simulink model "WheelLoads"  (R2020b)
settings: Solver=ode23  StartTime=0.0  StopTime=5  RelTol=0.01  AbsTol=0.01
PreLoadFcn:
  | addpath('Functions');
  | addpath('Functions/geometric');
  | addpath('Parameters');
  | vhclParams;
  | 
  | ELLIPSE = Simulink.Variant('variantMode == 1');
  | CONSTANT_ACC = Simulink.Variant('variantMode == 2');
  | CONSTANT_OUT = Simulink.Variant('variantMode == 3');
  | EXTERNAL_DATA_LOAD = Simulink.Variant('variantMode == 4');
  | EXTERNAL_DATA_ACC = Simulink.Variant('variantMode == 5');
  | if ~exist('F','var')
  |  F = 0;
  | end
  | if ~exist('M','var')
  |  M = 0;
  | end
  | if ~exist('St','var')
  |  St = 0;
  | end
  | if ~exist('Ax','var')
  |  Ax = 0;
  | end
  | if ~exist('Ay','var')
  |  Ay = 0;
  | end
  | if ~exist('StAcc','var')
  |  StAcc = 0;
  | end
InitFcn:
  | [folder, ~, ~] = fileparts(which (bdroot));
  | cd(folder);
undefined symbols (not defined in the file): ARB_bcrnk_frontL, ARB_bcrnk_frontR, ARB_bcrnk_rearL, ARB_bcrnk_rearR, ARB_center_front, ARB_center_rear, ARB_damping_front, ARB_damping_rear, ARB_length_front, ARB_length_rear, ARB_pinSlot_correctionFactor_front, ARB_pinSlot_correctionFactor_rear, ARB_stiffness_front, ARB_stiffness_rear, ARB_TieRod_length_front, ARB_TieRod_length_rear, COG, Damper_coefficient_front, Damper_coefficient_rear, Driver_COG, Driver_mass, DriveTrain_ratio, FS_camber, FS_toe, gravity, Mass, Rim_mass, RodDiameter, RodThickness, RS_camber, RS_toe, sep_lower_front, sep_lower_rear, sep_upper_front, sep_upper_rear, Spring_preload_front, Spring_preload_rear, Spring_stiffness_front, Spring_stiffness_rear, Sprung_mass, Sprung_mass_COG, sym_lower_front, sym_lower_rear, sym_upper_front, sym_upper_rear, Tire_mass, Tire_radius, Unsprung_mass_COG_front, Unsprung_mass_COG_rear, Unsprung_mass_front, Unsprung_mass_rear, util_rodCrossSection, Wheel_inertia, X, X_F, X_R, Y, Z
libraries: sm_lib x400, nesl_utility x70, fl_lib x1
(part 2 of 18)

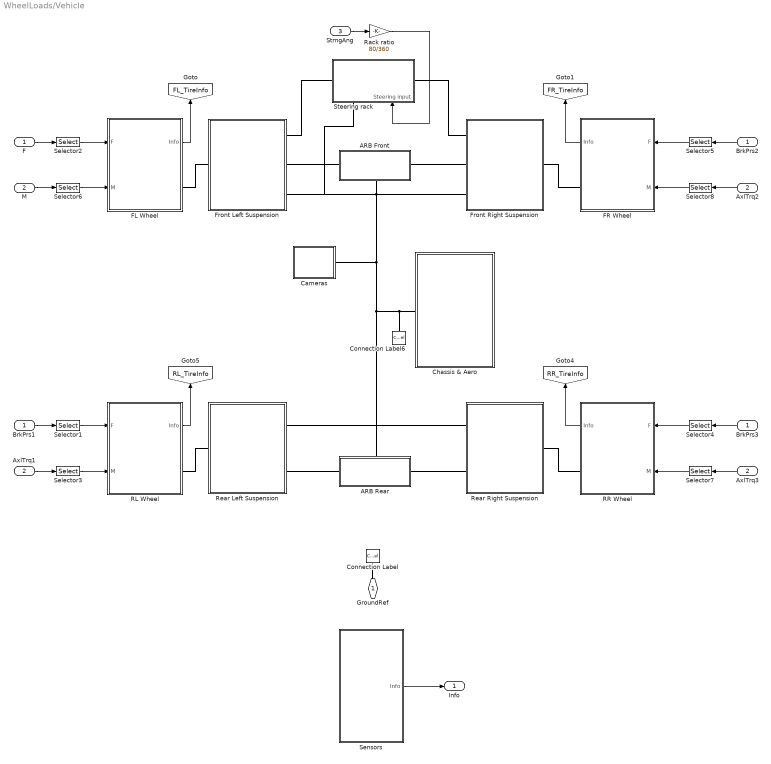
== system WheelLoads/Vehicle ==
  F : Inport
  M : Inport  Port=2
  StrngAng : Inport  Port=3
  ARB Front : Subsystem  mask{arbStiffness=ARB_stiffness_front, arbDamp=ARB_damping_front, arbCorrection=ARB_pinSlot_correctionFactor_front, arbCenter=ARB_center_front, arbLength=ARB_length_front, arbRodLength=ARB_TieRod_length_front, RodDiam=RodDiameter, RodThick=RodThickness}
      mask prompts: arbStiffness "Anti-roll Bar Stiffness"; arbDamp "Anti-roll Bar Damping"; arbCorrection "Stiffness correction factor"; arbCenter "Anti-roll Bar center"; arbLength "Anti-roll Bar length"; arbRodLength "Anti-roll Bar tie-rod length"; RodDiam "Rod diameter"; RodThick "Rod thickness"
      settings: PortSchema="{\"entries\":[{\"content\":{\"sides\":[{\"content\":{\"connectorIds\":[\"LConn1\"]},\"type\":\"ConnectorPlacement.EquallySpacedRectSide\",\"uuid\":\"7b39e7e2-1c99-4477-9d88-8155f0dcbcfa\"},{\"content\":{\"connectorIds\":[\"RConn1\"],\"side\":\"RIGHT\"},\"type\":\"ConnectorPlacement.EquallySpacedRectSide\",\"uuid\":\"e62feb70-34ca-4fb6-b792-0423ade04344\"},{\"content\":{\"connectorIds\":[],\"side\":\"TOP\"},\"type\":\"ConnectorPlacement.EquallySpacedRectSide\",\"uuid\":\"b26fb64d-892c-44a6-8f06-11f439d11cb6\"},{\"content\":{\"connectorIds\":[\"RConn2\"],\"side\":\"BOTTOM\"},\"type\":\"ConnectorPlacement.EquallySpacedRectSide\",\"uuid\":\"e4489596-2281-46d7-9d2e-4ef3bf65c061\"}]},\"type\":\"ConnectorPlacement.EquallySpacedConnector\",\"uuid\":\"4e7c908e-7a44-4075-b019-e55e6c824031\"}],\"packageUris\":[\"http://schema.mathworks.com/mf0/Connector/19700101\"],\"version\":\"1.0\"}"
      mask DialogControl DescGroupVar Prompt: Anti-roll Bar
      mask DialogControl DescTextVar Prompt: Z-Type anti-roll bar assembly
      mask DialogControl ParameterGroupVar Prompt: Dynamics
      mask DialogControl arbStiffness Tooltip: Anti-roll bar stiffness (N/mm)
      mask DialogControl arbDamp Tooltip: Anti-roll bar damping (N/(mm/s))
      mask DialogControl Container4 Prompt: Geometry
      mask DialogControl arbCenter Tooltip: Coordinates of the anti-roll bar center (mm)
      mask DialogControl arbLength Tooltip: Length of the anti-roll bar arm (mm)
      mask DialogControl arbRodLength Tooltip: length of the actuation rod of the anti-roll bar (mm)
      mask DialogControl Container5 Prompt: Graphic
      mask Display: image('$imagefile'); % Use "Edit Image" on toolstrip to change.
  ARB Rear : Subsystem  mask{arbStiffness=ARB_stiffness_rear, arbDamp=ARB_damping_rear, arbCorrection=ARB_pinSlot_correctionFactor_rear, arbCenter=ARB_center_rear, arbLength=ARB_length_rear, arbRodLength=ARB_TieRod_length_rear, RodDiam=RodDiameter, RodThick=RodThickness}
      mask prompts: arbStiffness "Anti-roll Bar Stiffness"; arbDamp "Anti-roll Bar Damping"; arbCorrection "Stiffness correction factor"; arbCenter "Anti-roll Bar center"; arbLength "Anti-roll Bar length"; arbRodLength "Anti-roll Bar tie-rod length"; RodDiam "Rod diameter"; RodThick "Rod thickness"
      settings: PortSchema="{\"entries\":[{\"content\":{\"sides\":[{\"content\":{\"connectorIds\":[\"LConn1\"]},\"type\":\"ConnectorPlacement.EquallySpacedRectSide\",\"uuid\":\"7b39e7e2-1c99-4477-9d88-8155f0dcbcfa\"},{\"content\":{\"connectorIds\":[\"RConn1\"],\"side\":\"RIGHT\"},\"type\":\"ConnectorPlacement.EquallySpacedRectSide\",\"uuid\":\"e62feb70-34ca-4fb6-b792-0423ade04344\"},{\"content\":{\"connectorIds\":[],\"side\":\"TOP\"},\"type\":\"ConnectorPlacement.EquallySpacedRectSide\",\"uuid\":\"b26fb64d-892c-44a6-8f06-11f439d11cb6\"},{\"content\":{\"connectorIds\":[\"RConn2\"],\"side\":\"BOTTOM\"},\"type\":\"ConnectorPlacement.EquallySpacedRectSide\",\"uuid\":\"e4489596-2281-46d7-9d2e-4ef3bf65c061\"}]},\"type\":\"ConnectorPlacement.EquallySpacedConnector\",\"uuid\":\"4e7c908e-7a44-4075-b019-e55e6c824031\"}],\"packageUris\":[\"http://schema.mathworks.com/mf0/Connector/19700101\"],\"version\":\"1.0\"}"
      mask DialogControl DescGroupVar Prompt: Anti-roll Bar
      mask DialogControl DescTextVar Prompt: Z-Type anti-roll bar assembly
      mask DialogControl ParameterGroupVar Prompt: Dynamics
      mask DialogControl arbStiffness Tooltip: Anti-roll bar stiffness (N/mm)
      mask DialogControl arbDamp Tooltip: Anti-roll bar damping (N/(mm/s))
      mask DialogControl Container4 Prompt: Geometry
      mask DialogControl arbCenter Tooltip: Coordinates of the anti-roll bar center (mm)
      mask DialogControl arbLength Tooltip: Length of the anti-roll bar arm (mm)
      mask DialogControl arbRodLength Tooltip: length of the actuation rod of the anti-roll bar (mm)
      mask DialogControl Container5 Prompt: Graphic
      mask Display: image('$imagefile'); % Use "Edit Image" on toolstrip to change.
  AxlTrq1 : InportShadow  Port=2
  AxlTrq2 : InportShadow  Port=2
  AxlTrq3 : InportShadow  Port=2
  BrkPrs1 : InportShadow
  BrkPrs2 : InportShadow
  BrkPrs3 : InportShadow
  Cameras : Subsystem
      mask Display: image('$imagefile'); % Use "Edit Image" on toolstrip to change.
  Chassis & Aero : Subsystem
      settings: PortSchema="{\"entries\":[{\"content\":{\"sides\":[{\"content\":{\"connectorIds\":[]},\"type\":\"ConnectorPlacement.EquallySpacedRectSide\",\"uuid\":\"cbbe6af3-19fb-48b3-8800-b215223b12f6\"},{\"content\":{\"connectorIds\":[],\"side\":\"RIGHT\"},\"type\":\"ConnectorPlacement.EquallySpacedRectSide\",\"uuid\":\"e0ce8cb6-c1e3-4be9-8f24-6f1308fe5f38\"},{\"content\":{\"connectorIds\":[\"LConn1\"],\"side\":\"TOP\"},\"type\":\"ConnectorPlacement.EquallySpacedRectSide\",\"uuid\":\"4e525be5-d309-4877-ab04-513b663981dd\"},{\"content\":{\"connectorIds\":[],\"side\":\"BOTTOM\"},\"type\":\"ConnectorPlacement.EquallySpacedRectSide\",\"uuid\":\"1a8d8adb-4793-4ada-a6b7-ecb65b96140c\"}]},\"type\":\"ConnectorPlacement.EquallySpacedConnector\",\"uuid\":\"0639ed7d-0262-43c4-ade7-52e76f974124\"}],\"packageUris\":[\"http://schema.mathworks.com/mf0/Connector/19700101\"],\"version\":\"1.0\"}"
      mask Display:
      | image('$imagefile'); % Use "Edit Image" on toolstrip to change.
      | port_label('LConn',1,'Ref')
  Connection Label : ConnectionLabel  Label=GroundRef
  Connection Label6 : ConnectionLabel  Label=GroundRef
  FL Wheel : Subsystem  mask{Wheel_mass=Rim_mass+Tire_mass, Wheel_inertia=Wheel_inertia, side=Left, sideSign=1}
      mask prompts: Wheel_mass "Wheel Mass"; Wheel_inertia "Wheel Inertia"
      mask TypeOptions side: Left | Right
      mask Callback side:
      | switch get_param(gcb,'side')
      |     case 'Left'
      |         set_param(gcb,'sideSign','1');
      |     case 'Right'
      |         set_param(gcb,'sideSign','-1');
      | end
      mask Display: image('$imagefile'); % Use "Edit Image" on toolstrip to change.
  FR Wheel : Subsystem  mask{Wheel_mass=Rim_mass+Tire_mass, Wheel_inertia=Wheel_inertia, side=Right, sideSign=-1}
      mask prompts: Wheel_mass "Wheel Mass"; Wheel_inertia "Wheel Inertia"
      mask TypeOptions side: Left | Right
      mask Callback side:
      | switch get_param(gcb,'side')
      |     case 'Left'
      |         set_param(gcb,'sideSign','1');
      |     case 'Right'
      |         set_param(gcb,'sideSign','-1');
      | end
      mask Display: image('$imagefile'); % Use "Edit Image" on toolstrip to change.
  Front Left Suspension : Subsystem  mask{X_original=X_F, X=[1090.2 243.1 189 810.3 257.6 186.1 1026.7 182.2 32.9 815.9 185.3 32.8 950.3 506 220 963.9 512 29 1090 225 42 1010 535 41 957 600 128.6 0 0.998629534754574 0.0523359562429438 950.3 487.586 237.194 955.54 278.351 490.117 951.389 225.774 445.625 953.389 219.438 514.305 950.3 40 499], enOptim=off, sepUpper=sep_upper_front, symUpper=sym_upper_front, sepLower=sep_lower_front, symLower=sym_lower_front, side=Left, sideSign=1, ARB_bcrnk=ARB_bcrnk_frontL, Camber=FS_camber, Toe=FS_toe, BcrnkPos=0, DamperPos=[0 90 0], ARB_On=on, St_On=on, SpringStiffness=Spring_stiffness_front, SpringPreload=Spring_preload_front, DamperCoef=Damper_coefficient_front, DamperMaxExt=200, DampLimit_MaxK=10000, DampLimit_MaxC=1, DampLimit_MaxTrans=1, DamperMinExt=154, DampLimit_MinK=2000, DampLimit_MinC=1, DampLimit_MinTrans=5, PushSetupLength=0, ToeSetupLength=0, UnsprungMass=Unsprung_mass_front-Tire_mass-Rim_mass, UnsprungMassCOG=Unsprung_mass_COG_front, RodThick=1, RodDiam=14, UCAfore_X=152.984798840791, UCAaft_X=-127.305532779834, UCA_Y=-257.3812, UCA_pushBracket_X=1.13049065291656, UCA_pushBracket_Y=-16.0659716079757, UCA_pushBracket_Z=19.373063236757, LCAfore_X=67.6445283762746, LCAaft_X=-143.178288225724, LCA_Y=-328.86366138683, UCA_Rot=[0.0129952164868874 0.0511310087922121 0.998607402480249;0.0511310087922121 0.997351198186899 -0.0517320733689305;-0.998607402480249 0.0517320733689305 0.0103464146737861], UCAfore_Rot=[-0.883968661732343 -0.0167990787372824 -0.467244257352307;-0.461720605527559 -0.125900009513931 0.878045141228887;-0.0735764059083774 0.991900689866667 0.103535182115236], UCAaft_Rot=[-0.872927868673978 -0.0167990787372824 0.48755997276836;0.485594358546025 -0.125900009513931 0.865070694540433;0.0468514144992482 0.991900689866667 0.118059164834624], LCA_Rot=[0.000690445618848567 0.0146957287408994 0.99989177356433;0.0146957287408994 0.999783886342046 -0.0147042907877108;-0.99989177356433 0.0147042907877108 0.000474331960894192], LCAfore_Rot=[-0.982351350121625 -0.000301304030408464 -0.187044738311724;-0.187035279606844 0.0117671555848941 0.982282718076538;0.00190501879509281 0.999930719232749 -0.011615835659486], LCAaft_Rot=[-0.91090135192941 -0.000301304030408464 0.412624085905142;0.412592306855799 0.0117671555848941 0.910839789629796;-0.00512985151659236 0.999930719232749 -0.0105944022056725], BcrnkDamp=69.0006325768104, BcrnkPush=69.0007956041088, BcrnkAng=54.9995482296285, BcrnkRot=[-0.0310893815864683 0.0519417534127774 0.998166070654066;0.0519417534127774 0.997383402646003 -0.0502832216418927;-0.998166070654066 0.0502832216418927 -0.0337059789404655], PushRot=[0.998890321638943 0.0443098381442263 -0.0159613150987419;0.0443098381442263 -0.769307058035718 0.637340794787264;0.0159613150987419 -0.637340794787264 -0.770416736396775], SteeringRot=[0.937365949814546 0.242706944468636 -0.249876800113523;0.242706944468636 0.0595105901840355 0.9682726004399;0.249876800113523 -0.9682726004399 -0.00312346000141894], Parameter55=on, Parameter56=0}
      mask prompts: X_original "Coordinates"; X "Coordinates_"; enOptim "Enable"; sepUpper "Separation"; symUpper "Symmetry"; sepLower "Separation"; symLower "Symmety"; ARB_bcrnk "ARB tie-rod"; BcrnkPos "Bellcrank initial rotation"; DamperPos "Damper initial position"; ARB_On "Anti-roll bar"; St_On "Steering"; SpringStiffness "Spring stiffness"; SpringPreload "Spring preload"; DamperCoef "Damping Coefficient"; DamperMaxExt "Maximum extension"; DampLimit_MaxK "Max_Stiffness"; DampLimit_MaxC "Max_Damping"; DampLimit_MaxTrans "Max_Transition"; DamperMinExt "Maximum compression"; DampLimit_MinK "Min_Stiffness"; DampLimit_MinC "Min_Damping"; DampLimit_MinTrans "Min_Transition"; PushSetupLength "Push"; ToeSetupLength "Toe"; UnsprungMass "Mass"; UnsprungMassCOG "COG"; RodThick "Rod Thickness"; RodDiam "Rod Diameter"
      mask help: Define a Simscape Multibody model of a double control arm independent suspension
      mask init:
      | dblAarmSuspOptim_init(gcb)
      mask Help:
      | -Geometry:
      |     +Suspension Coordinates: coordinates of the points that define a double wishbone suspension system. The coordinates must always correspond to the LEFT side. The variable (e.g. 'X') containing the data must be a row vector with the following values, in order:
      |       1-A-Arm Upper Front hardpoint. X([1 2 3])
      |       2-A-Arm Upper Rear. X([4 5 6])
      |       3-A-Arm Lower Front. X([7 8 9])
      |       4-A-Arm Lower Rear. X([10 11 12])
      |       5-A-Arm Upper Upright. X([13 14 15])
      |       6-A-Arm Lower Upright. X([16 17 18])
      |       7-Steering Rack. X([19 20 21])
      |       8-Steering Upright. X([22 23 24])
      |       9-Wheel Center. X([25 26 27])
      |       10-Wheel Plane normal vector. X([28 29 30])
      |       11-A-Arm Push Bracket. X([31 32 33])
      |       12-Bellcrank Push Bracket. X([34 35 36])
      |       13-Bellcrank Chassis Bracket. X([37 38 39])
      |       14-Bellcrank Damper Bracket. X([40 41 42])
      |       15-Damper Bracket. X([43 44 45])
      |       16-Bellcrank ARB Bracket. X(46:48)
      |       17-ARB end. X(49:51)
      |       18-ARB center. X(52:54)
      |     +It must be noted that in the model the bellcranks have the same dimensions as defined in the geometry, but the position and orientation is diferent. It is positioned in a vertical plane that contains the a-arm push bracket. The reason is to let the anti-roll bar be symmetric.
      mask TypeOptions side: Left | Right
      mask Callback side:
      | switch get_param(gcb,'side')
      |     case 'Left'
      |         set_param(gcb,'sideSign','1');
      |     case 'Right'
      |         set_param(gcb,'sideSign','-1');
      | end
      mask DialogControl DescGroupVar Prompt: Double Wishbone Suspension
      mask DialogControl SuspGeomTab Prompt: Geometry
      mask DialogControl X_original Tooltip: Variable containting suspension points coordinates
      mask DialogControl Container25 Prompt: Modify a-arms geometry
      mask DialogControl enOptim Tooltip: Below parameters are used to modify the provided geometry. In this enable button is off no changes will be made
      mask DialogControl Container26 Prompt: Upper arm
      mask DialogControl sepUpper Tooltip: Separation between a-arm hardpoints (mm)
      mask DialogControl symUpper Tooltip: Symmetry factor. Value between -1 and 1. Use 0 for perfectly symmetric arm
      mask DialogControl Container27 Prompt: Lower arm
      mask DialogControl sepLower Tooltip: Separation between a-arm hardpoints (mm)
      mask DialogControl symLower Tooltip: Symmetry factor. Value between -1 and 1. Use 0 for perfectly symmetric arm
      mask DialogControl Container22 Prompt: Anti-roll Bar
      mask DialogControl ARB_bcrnk Tooltip: Coordinates of the attachment point of the ARB to the bellcrank (mm)
      mask DialogControl Container8 Prompt: Wheel angles
      mask DialogControl Camber Tooltip: Camber angle in deg at reference ride height
      mask DialogControl Toe Tooltip: Toe angle in deg at reference ride height
      mask DialogControl Container23 Prompt: Assembly
      mask DialogControl BcrnkPos Tooltip: Degrees of rotation of follower frame respect to base frame of the bellcrank bracket joint for the inital position
      mask DialogControl DamperPos Tooltip: Degrees of rotation of follower frame respect to base frame of the bellcrank bracket joint for the inital position, specified as a rotation sequence X-Y-Z
      mask DialogControl ARB_On Tooltip: Select wheter the block displays connection for ARB or not
      mask DialogControl St_On Tooltip: Select wether the block displays steering input or not
      mask DialogControl SpringDampTab Prompt: Spring and Damper
      mask DialogControl SpringStiffness Tooltip: Spring stiffness (lb/inch)
      mask DialogControl SpringPreload Tooltip: Spring preload in mm
      mask DialogControl DamperCoef Tooltip: Damping Coefficient in N/(mm/s)
      mask DialogControl Container9 Prompt: Limits
      mask DialogControl DamperMaxExt Tooltip: Maximum allowable extension of the damper, meassured between ball-joints. This value is also used for the natural length of the spring (mm)
      mask DialogControl DampLimit_MaxK Tooltip: Stiffness when upper limit is reached in N/mm
      mask DialogControl DampLimit_MaxC Tooltip: Damping coefficient when upper limit is reached in N/(mm/s)
      mask DialogControl DampLimit_MaxTrans Tooltip: Transition region width between normal behavior and limit
      mask DialogControl DamperMinExt Tooltip: Minimum allowable extension (max compression) of the damper, meassured between ball-joints (mm)
      mask DialogControl DampLimit_MinK Tooltip: Stiffness when lower limit is reached in N/(mm/s)
      mask DialogControl DampLimit_MinC Tooltip: Damping coefficient when lower limit is reached in N/(mm/s)
      mask DialogControl DampLimit_MinTrans Tooltip: Transition region width between normal behavior and limit
      mask DialogControl Container13 Prompt: Setup
      mask DialogControl PushSetupLength Tooltip: Length adjustment of the rod ends in the push rod
      mask DialogControl ToeSetupLength Tooltip: Length adjustment of the rod ends in the toe/steering rod
      mask DialogControl Container19 Prompt: Mass
      mask DialogControl Container20 Prompt: Non-rotating unsprung mass
      mask DialogControl UnsprungMass Tooltip: Non rotating unsprung mass (kg)
      mask DialogControl UnsprungMassCOG Tooltip: Non-rotating unsprung mass center of gravity coordinates (mm)
      mask DialogControl Container17 Prompt: Graphic
      mask DialogControl internalParamContainer Prompt: Internal params
      mask Display: image('$imagefile'); % Use "Edit Image" on toolstrip to change.
  Front Right Suspension : Subsystem  mask{X_original=X_F, X=[1090.2 243.1 189 810.3 257.6 186.1 1026.7 182.2 32.9 815.9 185.3 32.8 950.3 506 220 963.9 512 29 1090 225 42 1010 535 41 957 600 128.6 0 0.998629534754574 0.0523359562429438 950.3 487.586 237.194 955.54 278.351 490.117 951.389 225.774 445.625 953.389 219.438 514.305 950.3 40 499], enOptim=off, sepUpper=sep_upper_front, symUpper=sym_upper_front, sepLower=sep_lower_front, symLower=sym_lower_front, side=Right, sideSign=-1, ARB_bcrnk=ARB_bcrnk_frontR, Camber=FS_camber, Toe=FS_toe, BcrnkPos=0, DamperPos=[0 90 0], ARB_On=on, St_On=on, SpringStiffness=Spring_stiffness_front, SpringPreload=Spring_preload_front, DamperCoef=Damper_coefficient_front, DamperMaxExt=200, DampLimit_MaxK=10000, DampLimit_MaxC=1, DampLimit_MaxTrans=1, DamperMinExt=154, DampLimit_MinK=2000, DampLimit_MinC=1, DampLimit_MinTrans=5, PushSetupLength=0, ToeSetupLength=0, UnsprungMass=Unsprung_mass_front-Tire_mass-Rim_mass, UnsprungMassCOG=Unsprung_mass_COG_front, RodThick=1, RodDiam=14, UCAfore_X=152.984798840791, UCAaft_X=-127.305532779834, UCA_Y=-257.3812, UCA_pushBracket_X=1.13049065291656, UCA_pushBracket_Y=-16.0659716079757, UCA_pushBracket_Z=19.373063236757, LCAfore_X=67.6445283762746, LCAaft_X=-143.178288225724, LCA_Y=-328.86366138683, UCA_Rot=[0.0129952164868874 -0.0511310087922121 0.998607402480249;-0.0511310087922121 0.997351198186899 0.0517320733689305;-0.998607402480249 -0.0517320733689305 0.0103464146737861], UCAfore_Rot=[-0.883968661732343 0.0167990787372824 -0.467244257352307;0.461720605527559 -0.125900009513931 -0.878045141228887;-0.0735764059083774 -0.991900689866667 0.103535182115236], UCAaft_Rot=[-0.872927868673978 0.0167990787372824 0.48755997276836;-0.485594358546025 -0.125900009513931 -0.865070694540433;0.0468514144992482 -0.991900689866667 0.118059164834624], LCA_Rot=[0.000690445618848567 -0.0146957287408994 0.99989177356433;-0.0146957287408994 0.999783886342046 0.0147042907877108;-0.99989177356433 -0.0147042907877108 0.000474331960894192], LCAfore_Rot=[-0.982351350121625 0.000301304030408464 -0.187044738311724;0.187035279606844 0.0117671555848941 -0.982282718076538;0.00190501879509281 -0.999930719232749 -0.011615835659486], LCAaft_Rot=[-0.91090135192941 0.000301304030408464 0.412624085905142;-0.412592306855799 0.0117671555848941 -0.910839789629796;-0.00512985151659236 -0.999930719232749 -0.0105944022056725], BcrnkDamp=69.0006325768104, BcrnkPush=69.0007956041088, BcrnkAng=54.9995482296285, BcrnkRot=[-0.0310893815864683 -0.0519417534127774 0.998166070654066;-0.0519417534127774 0.997383402646003 0.0502832216418927;-0.998166070654066 -0.0502832216418927 -0.0337059789404655], PushRot=[0.998890321638943 -0.0443098381442263 -0.0159613150987419;-0.0443098381442263 -0.769307058035718 -0.637340794787264;0.0159613150987419 0.637340794787264 -0.770416736396775], SteeringRot=[0.937365949814546 -0.242706944468636 -0.249876800113523;-0.242706944468636 0.0595105901840355 -0.9682726004399;0.249876800113523 0.9682726004399 -0.00312346000141894]}
      mask prompts: X_original "Coordinates"; X "Coordinates_"; enOptim "Enable"; sepUpper "Separation"; symUpper "Symmetry"; sepLower "Separation"; symLower "Symmety"; ARB_bcrnk "ARB tie-rod"; BcrnkPos "Bellcrank initial rotation"; DamperPos "Damper initial position"; ARB_On "Anti-roll bar"; St_On "Steering"; SpringStiffness "Spring stiffness"; SpringPreload "Spring preload"; DamperCoef "Damping Coefficient"; DamperMaxExt "Maximum extension"; DampLimit_MaxK "Max_Stiffness"; DampLimit_MaxC "Max_Damping"; DampLimit_MaxTrans "Max_Transition"; DamperMinExt "Maximum compression"; DampLimit_MinK "Min_Stiffness"; DampLimit_MinC "Min_Damping"; DampLimit_MinTrans "Min_Transition"; PushSetupLength "Push"; ToeSetupLength "Toe"; UnsprungMass "Mass"; UnsprungMassCOG "COG"; RodThick "Rod Thickness"; RodDiam "Rod Diameter"
      mask help: Define a Simscape Multibody model of a double control arm independent suspension
      mask init:
      | dblAarmSuspOptim_init(gcb)
      mask Help:
      | -Geometry:
      |     +Suspension Coordinates: coordinates of the points that define a double wishbone suspension system. The coordinates must always correspond to the LEFT side. The variable (e.g. 'X') containing the data must be a row vector with the following values, in order:
      |       1-A-Arm Upper Front hardpoint. X([1 2 3])
      |       2-A-Arm Upper Rear. X([4 5 6])
      |       3-A-Arm Lower Front. X([7 8 9])
      |       4-A-Arm Lower Rear. X([10 11 12])
      |       5-A-Arm Upper Upright. X([13 14 15])
      |       6-A-Arm Lower Upright. X([16 17 18])
      |       7-Steering Rack. X([19 20 21])
      |       8-Steering Upright. X([22 23 24])
      |       9-Wheel Center. X([25 26 27])
      |       10-Wheel Plane normal vector. X([28 29 30])
      |       11-A-Arm Push Bracket. X([31 32 33])
      |       12-Bellcrank Push Bracket. X([34 35 36])
      |       13-Bellcrank Chassis Bracket. X([37 38 39])
      |       14-Bellcrank Damper Bracket. X([40 41 42])
      |       15-Damper Bracket. X([43 44 45])
      |       16-Bellcrank ARB Bracket. X(46:48)
      |       17-ARB end. X(49:51)
      |       18-ARB center. X(52:54)
      |     +It must be noted that in the model the bellcranks have the same dimensions as defined in the geometry, but the position and orientation is diferent. It is positioned in a vertical plane that contains the a-arm push bracket. The reason is to let the anti-roll bar be symmetric.
      mask TypeOptions side: Left | Right
      mask Callback side:
      | switch get_param(gcb,'side')
      |     case 'Left'
      |         set_param(gcb,'sideSign','1');
      |     case 'Right'
      |         set_param(gcb,'sideSign','-1');
      | end
      mask DialogControl DescGroupVar Prompt: Double Wishbone Suspension
      mask DialogControl SuspGeomTab Prompt: Geometry
      mask DialogControl X_original Tooltip: Variable containting suspension points coordinates
      mask DialogControl Container25 Prompt: A-arms geometry
      mask DialogControl enOptim Tooltip: Below parameters are used to modify the provided geometry. In this enable button is off no changes will be made
      mask DialogControl Container26 Prompt: Upper arm
      mask DialogControl sepUpper Tooltip: Separation between a-arm hardpoints (mm)
      mask DialogControl symUpper Tooltip: Symmetry factor. Value between -1 and 1. Use 0 for perfectly symmetric arm
      mask DialogControl Container27 Prompt: Lower arm
      mask DialogControl sepLower Tooltip: Separation between a-arm hardpoints (mm)
      mask DialogControl symLower Tooltip: Symmetry factor. Value between -1 and 1. Use 0 for perfectly symmetric arm
      mask DialogControl Container22 Prompt: Anti-roll Bar
      mask DialogControl ARB_bcrnk Tooltip: Coordinates of the attachment point of the ARB to the bellcrank (mm)
      mask DialogControl Container8 Prompt: Wheel angles
      mask DialogControl Camber Tooltip: Camber angle in deg at reference ride height
      mask DialogControl Toe Tooltip: Toe angle in deg at reference ride height
      mask DialogControl Container23 Prompt: Assembly
      mask DialogControl BcrnkPos Tooltip: Degrees of rotation of follower frame respect to base frame of the bellcrank bracket joint for the inital position
      mask DialogControl DamperPos Tooltip: Degrees of rotation of follower frame respect to base frame of the bellcrank bracket joint for the inital position, specified as a rotation sequence X-Y-Z
      mask DialogControl ARB_On Tooltip: Select wheter the block displays connection for ARB or not
      mask DialogControl St_On Tooltip: Select wether the block displays steering input or not
      mask DialogControl SpringDampTab Prompt: Spring and Damper
      mask DialogControl SpringStiffness Tooltip: Spring stiffness (lb/inch)
      mask DialogControl SpringPreload Tooltip: Spring preload in mm
      mask DialogControl DamperCoef Tooltip: Damping Coefficient in N/(mm/s)
      mask DialogControl Container9 Prompt: Limits
      mask DialogControl DamperMaxExt Tooltip: Maximum allowable extension of the damper, meassured between ball-joints. This value is also used for the natural length of the spring (mm)
      mask DialogControl DampLimit_MaxK Tooltip: Stiffness when upper limit is reached in N/mm
      mask DialogControl DampLimit_MaxC Tooltip: Damping coefficient when upper limit is reached in N/(mm/s)
      mask DialogControl DampLimit_MaxTrans Tooltip: Transition region width between normal behavior and limit
      mask DialogControl DamperMinExt Tooltip: Minimum allowable extension (max compression) of the damper, meassured between ball-joints (mm)
      mask DialogControl DampLimit_MinK Tooltip: Stiffness when lower limit is reached in N/(mm/s)
      mask DialogControl DampLimit_MinC Tooltip: Damping coefficient when lower limit is reached in N/(mm/s)
      mask DialogControl DampLimit_MinTrans Tooltip: Transition region width between normal behavior and limit
      mask DialogControl Container13 Prompt: Setup
      mask DialogControl PushSetupLength Tooltip: Length adjustment of the rod ends in the push rod
      mask DialogControl ToeSetupLength Tooltip: Length adjustment of the rod ends in the toe/steering rod
      mask DialogControl Container19 Prompt: Mass
      mask DialogControl Container20 Prompt: Non-rotating unsprung mass
      mask DialogControl UnsprungMass Tooltip: Non rotating unsprung mass (kg)
      mask DialogControl UnsprungMassCOG Tooltip: Non-rotating unsprung mass center of gravity coordinates (mm)
      mask DialogControl Container17 Prompt: Graphic
      mask DialogControl internalParamContainer Prompt: Internal params
      mask Display: image('$imagefile'); % Use "Edit Image" on toolstrip to change.
  RL Wheel : Subsystem  mask{Wheel_mass=Rim_mass+Tire_mass, Wheel_inertia=Wheel_inertia, side=Left, sideSign=1}
      mask prompts: Wheel_mass "Wheel Mass"; Wheel_inertia "Wheel Inertia"
      mask TypeOptions side: Left | Right
      mask Callback side:
      | switch get_param(gcb,'side')
      |     case 'Left'
      |         set_param(gcb,'sideSign','1');
      |     case 'Right'
      |         set_param(gcb,'sideSign','-1');
      | end
      mask Display: image('$imagefile'); % Use "Edit Image" on toolstrip to change.
  RR Wheel : Subsystem  mask{Wheel_mass=Rim_mass+Tire_mass, Wheel_inertia=Wheel_inertia, side=Right, sideSign=-1}
      mask prompts: Wheel_mass "Wheel Mass"; Wheel_inertia "Wheel Inertia"
      mask TypeOptions side: Left | Right
      mask Callback side:
      | switch get_param(gcb,'side')
      |     case 'Left'
      |         set_param(gcb,'sideSign','1');
      |     case 'Right'
      |         set_param(gcb,'sideSign','-1');
      | end
      mask Display: image('$imagefile'); % Use "Edit Image" on toolstrip to change.
  Rack ratio : Gain  Gain=80/360
  Rear Left Suspension : Subsystem  mask{X_original=X_R, X=[-463.9 281.7 173.2 -726.9 257.8 175.9 -425.3 210.1 30 -731.2 210.1 30 -598 515 220 -593 539 30 -684.7 212.3 71.5 -690 535 90 -593 600 128.6 0 0.998629534754574 0.0523359562429438 -598 494.706 234.934 -601.182370576922 286.101220010582 418.414652998722 -598 242.526 367.561 -601.131598670298 215.919391219277 442.981059527181 -598 40 405], enOptim=off, sepUpper=sep_upper_rear, symUpper=sym_upper_rear, sepLower=sep_lower_rear, symLower=sym_lower_rear, side=Left, sideSign=1, ARB_bcrnk=ARB_bcrnk_rearL, Camber=RS_camber, Toe=RS_toe, BcrnkPos=0, DamperPos=[0 90 0], ARB_On=on, St_On=on, SpringStiffness=Spring_stiffness_rear, SpringPreload=Spring_preload_rear, DamperCoef=Damper_coefficient_rear, DamperMaxExt=200, DampLimit_MaxK=10000, DampLimit_MaxC=1, DampLimit_MaxTrans=1, DamperMinExt=154, DampLimit_MinK=2000, DampLimit_MinC=1, DampLimit_MinTrans=5, PushSetupLength=0, ToeSetupLength=0, UnsprungMass=Unsprung_mass_rear-Tire_mass-Rim_mass, UnsprungMassCOG=Unsprung_mass_COG_rear, RodThick=1, RodDiam=14, UCAfore_X=112.908254553074, UCAaft_X=-151.189265314115, UCA_Y=-248.704, UCA_pushBracket_X=-1.98922125533095, UCA_pushBracket_Y=-17.1299173592706, UCA_pushBracket_Z=18.3706211670128, LCAfore_X=167.7, LCAaft_X=-138.2, LCA_Y=-328.9, UCA_Rot=[-0.00194921998470532 -0.0910516591545036 0.995844262877817;-0.0910516591545036 0.991725723749838 0.0904968740790107;-0.995844262877817 -0.0904968740790107 -0.0102234962348675], UCAfore_Rot=[-0.870770481943406 0.0266195803215717 -0.490968599525011;-0.487163699387331 -0.181881053850026 0.854160881947689;-0.0665604820843295 0.982960162058416 0.171344746142957], UCAaft_Rot=[-0.896188291915812 0.0266195803215717 0.442874636185596;0.431294757674712 -0.181881053850026 0.883687792295851;0.104073903719095 0.982960162058416 0.151518785537508], LCA_Rot=[0 0 1;0 1 0;-1 0 0], LCAfore_Rot=[-0.890878207254611 0 -0.454242247967766;-0.454242247967766 0 0.890878207254611;0 1 0], LCAaft_Rot=[-0.921920047549027 0 0.3873802084867;0.3873802084867 0 0.921920047549027;0 1 0], BcrnkDamp=80.0368909948768, BcrnkPush=67.0449200519613, BcrnkAng=59.9638794672988, BcrnkRot=[0.047890311837444 -0.016573734999306 0.998715089172225;-0.016573734999306 0.999711494699358 0.0173850129176741;-0.998715089172225 -0.0173850129176741 0.0476018065368023], PushRot=[0.999613664741765 -0.0253243233615474 0.0114542528266899;-0.0253243233615474 -0.660012489282967 0.750827671730858;-0.0114542528266899 -0.750827671730858 -0.660398824541202], SteeringRot=[0.999745760072107 0.0154798537228226 -0.0163947963257976;0.0154798537228226 0.0574813591783186 0.99822656119527;0.0163947963257976 -0.99822656119527 0.057227119250426]}
      mask prompts: X_original "Coordinates"; X "Coordinates_"; enOptim "Enable"; sepUpper "Separation"; symUpper "Symmetry"; sepLower "Separation"; symLower "Symmetry"; ARB_bcrnk "ARB tie-rod"; BcrnkPos "Bellcrank initial rotation"; DamperPos "Damper initial position"; ARB_On "Anti-roll bar"; St_On "Steering"; SpringStiffness "Spring stiffness"; SpringPreload "Spring preload"; DamperCoef "Damping Coefficient"; DamperMaxExt "Maximum extension"; DampLimit_MaxK "Max_Stiffness"; DampLimit_MaxC "Max_Damping"; DampLimit_MaxTrans "Max_Transition"; DamperMinExt "Maximum compression"; DampLimit_MinK "Min_Stiffness"; DampLimit_MinC "Min_Damping"; DampLimit_MinTrans "Min_Transition"; PushSetupLength "Push"; ToeSetupLength "Toe"; UnsprungMass "Mass"; UnsprungMassCOG "COG"; RodThick "Rod Thickness"; RodDiam "Rod Diameter"
      mask help: Define a Simscape Multibody model of a double control arm independent suspension
      mask init:
      | dblAarmSuspOptim_init(gcb)
      mask Help:
      | -Geometry:
      |     +Suspension Coordinates: coordinates of the points that define a double wishbone suspension system. The coordinates must always correspond to the LEFT side. The variable (e.g. 'X') containing the data must be a row vector with the following values, in order:
      |       1-A-Arm Upper Front hardpoint. X([1 2 3])
      |       2-A-Arm Upper Rear. X([4 5 6])
      |       3-A-Arm Lower Front. X([7 8 9])
      |       4-A-Arm Lower Rear. X([10 11 12])
      |       5-A-Arm Upper Upright. X([13 14 15])
      |       6-A-Arm Lower Upright. X([16 17 18])
      |       7-Steering Rack. X([19 20 21])
      |       8-Steering Upright. X([22 23 24])
      |       9-Wheel Center. X([25 26 27])
      |       10-Wheel Plane normal vector. X([28 29 30])
      |       11-A-Arm Push Bracket. X([31 32 33])
      |       12-Bellcrank Push Bracket. X([34 35 36])
      |       13-Bellcrank Chassis Bracket. X([37 38 39])
      |       14-Bellcrank Damper Bracket. X([40 41 42])
      |       15-Damper Bracket. X([43 44 45])
      |       16-Bellcrank ARB Bracket. X(46:48)
      |       17-ARB end. X(49:51)
      |       18-ARB center. X(52:54)
      |     +It must be noted that in the model the bellcranks have the same dimensions as defined in the geometry, but the position and orientation is diferent. It is positioned in a vertical plane that contains the a-arm push bracket. The reason is to let the anti-roll bar be symmetric.
      | 
      | -Graphic: a CAD file can be specified to graphically represent some parts of the suspension. In order for the part to appear in its proper position, its reference axis and origin in the CAD file must be the same as the reference for the suspension points coordinates.
      mask TypeOptions side: Left | Right
      mask Callback side:
      | switch get_param(gcb,'side')
      |     case 'Left'
      |         set_param(gcb,'sideSign','1');
      |     case 'Right'
      |         set_param(gcb,'sideSign','-1');
      | end
      mask DialogControl DescGroupVar Prompt: Double Wishbone Suspension
      mask DialogControl SuspGeomTab Prompt: Geometry
      mask DialogControl X_original Tooltip: Variable containting suspension points coordinates
      mask DialogControl Container25 Prompt: A-arms geometry
      mask DialogControl enOptim Tooltip: Below parameters are used to modify the provided geometry. In this enable button is off no changes will be made
      mask DialogControl Container26 Prompt: Upper arm
      mask DialogControl Container27 Prompt: Lower arm
      mask DialogControl Container23 Prompt: Anti-roll bar
      mask DialogControl ARB_bcrnk Tooltip: Coordinates of the attachment point of the anti-roll bar to the bellcrank (mm)
      mask DialogControl Container8 Prompt: Wheel angles
      mask DialogControl Camber Tooltip: Camber angle in deg at reference ride height
      mask DialogControl Toe Tooltip: Toe angle in deg at reference ride height
      mask DialogControl Container22 Prompt: Assembly
      mask DialogControl BcrnkPos Tooltip: Degrees of rotation of follower frame respect to base frame of the bellcrank bracket joint for the inital position
      mask DialogControl DamperPos Tooltip: Degrees of rotation of follower frame respect to base frame of the bellcrank bracket joint for the inital position, specified as a rotation sequence X-Y-Z
      mask DialogControl ARB_On Tooltip: Select wheter the block displays connection for ARB or not
      mask DialogControl St_On Tooltip: Select wether the block displays steering input or not
      mask DialogControl SpringDampTab Prompt: Spring and Damper
      mask DialogControl SpringStiffness Tooltip: Spring stiffness in lb/inch
      mask DialogControl SpringPreload Tooltip: Spring preload in mm
      mask DialogControl DamperCoef Tooltip: Damping Coefficient in N/(mm/s)
      mask DialogControl Container9 Prompt: Limits
      mask DialogControl DamperMaxExt Tooltip: Maximum allowable extension of the damper, meassured between ball-joints (mm)
      mask DialogControl DampLimit_MaxK Tooltip: Stiffness when upper limit is reached in N/mm
      mask DialogControl DampLimit_MaxC Tooltip: Damping coefficient when upper limit is reached in N/(mm/s)
      mask DialogControl DampLimit_MaxTrans Tooltip: Transition region width between normal behavior and limit
      mask DialogControl DamperMinExt Tooltip: Minimum allowable extension (max compression) of the damper, meassured between ball-joints (mm)
      mask DialogControl DampLimit_MinK Tooltip: Stiffness when lower limit is reached in N/(mm/s)
      mask DialogControl DampLimit_MinC Tooltip: Damping coefficient when lower limit is reached in N/(mm/s)
      mask DialogControl DampLimit_MinTrans Tooltip: Transition region width between normal behavior and limit
      mask DialogControl Container13 Prompt: Setup
      mask DialogControl PushSetupLength Tooltip: Length adjustment of the rod ends in the push rod
      mask DialogControl ToeSetupLength Tooltip: Length adjustment of the rod ends in the toe/steering rod
      mask DialogControl Container19 Prompt: Mass
      mask DialogControl Container20 Prompt: Non-rotating unsprung mass
      mask DialogControl UnsprungMass Tooltip: Non rotating unsprung mass (kg)
      mask DialogControl UnsprungMassCOG Tooltip: Non-rotating unsprung mass center of gravity coordinates (mm)
      mask DialogControl Container17 Prompt: Graphic
      mask DialogControl internalParamContainer Prompt: Internal params
      mask Display: image('$imagefile'); % Use "Edit Image" on toolstrip to change.
  Rear Right Suspension : Subsystem  mask{X_original=X_R, X=[-463.9 281.7 173.2 -726.9 257.8 175.9 -425.3 210.1 30 -731.2 210.1 30 -598 515 220 -593 539 30 -684.7 212.3 71.5 -690 535 90 -593 600 128.6 0 0.998629534754574 0.0523359562429438 -598 494.706 234.934 -601.182370576922 286.101220010582 418.414652998722 -598 242.526 367.561 -601.131598670298 215.919391219277 442.981059527181 -598 40 405], enOptim=off, sepUpper=sep_upper_rear, symUpper=sym_upper_rear, sepLower=sep_lower_rear, symLower=sym_lower_rear, side=Right, sideSign=-1, ARB_bcrnk=ARB_bcrnk_rearR, Camber=RS_camber, Toe=RS_toe, BcrnkPos=0, DamperPos=[0 90 0], ARB_On=on, St_On=on, SpringStiffness=Spring_stiffness_rear, SpringPreload=Spring_preload_rear, DamperCoef=Damper_coefficient_rear, DamperMaxExt=200, DampLimit_MaxK=10000, DampLimit_MaxC=1, DampLimit_MaxTrans=1, DamperMinExt=154, DampLimit_MinK=2000, DampLimit_MinC=1, DampLimit_MinTrans=5, PushSetupLength=0, ToeSetupLength=0, UnsprungMass=Unsprung_mass_rear-Tire_mass-Rim_mass, UnsprungMassCOG=Unsprung_mass_COG_rear, RodThick=1, RodDiam=14, UCAfore_X=112.908254553074, UCAaft_X=-151.189265314115, UCA_Y=-248.704, UCA_pushBracket_X=-1.98922125533095, UCA_pushBracket_Y=-17.1299173592706, UCA_pushBracket_Z=18.3706211670128, LCAfore_X=167.7, LCAaft_X=-138.2, LCA_Y=-328.9, UCA_Rot=[-0.00194921998470532 0.0910516591545036 0.995844262877817;0.0910516591545036 0.991725723749838 -0.0904968740790107;-0.995844262877817 0.0904968740790107 -0.0102234962348675], UCAfore_Rot=[-0.870770481943406 -0.0266195803215717 -0.490968599525011;0.487163699387331 -0.181881053850026 -0.854160881947689;-0.0665604820843295 -0.982960162058416 0.171344746142957], UCAaft_Rot=[-0.896188291915812 -0.0266195803215717 0.442874636185596;-0.431294757674712 -0.181881053850026 -0.883687792295851;0.104073903719095 -0.982960162058416 0.151518785537508], LCA_Rot=[0 0 1;0 1 0;-1 0 0], LCAfore_Rot=[-0.890878207254611 0 -0.454242247967766;0.454242247967766 0 -0.890878207254611;0 -1 0], LCAaft_Rot=[-0.921920047549027 0 0.3873802084867;-0.3873802084867 0 -0.921920047549027;0 -1 0], BcrnkDamp=80.0368909948768, BcrnkPush=67.0449200519613, BcrnkAng=59.9638794672988, BcrnkRot=[0.047890311837444 0.016573734999306 0.998715089172225;0.016573734999306 0.999711494699358 -0.0173850129176741;-0.998715089172225 0.0173850129176741 0.0476018065368023], PushRot=[0.999613664741765 0.0253243233615474 0.0114542528266899;0.0253243233615474 -0.660012489282967 -0.750827671730858;-0.0114542528266899 0.750827671730858 -0.660398824541202], SteeringRot=[0.999745760072107 -0.0154798537228226 -0.0163947963257976;-0.0154798537228226 0.0574813591783186 -0.99822656119527;0.0163947963257976 0.99822656119527 0.057227119250426]}
      mask prompts: X_original "Coordinates"; X "Coordinates_"; enOptim "Enable"; sepUpper "Separation"; symUpper "Symmetry"; sepLower "Separation"; symLower "Symmetry"; ARB_bcrnk "ARB tie-rod"; BcrnkPos "Bellcrank initial rotation"; DamperPos "Damper initial position"; ARB_On "Anti-roll bar"; St_On "Steering"; SpringStiffness "Spring stiffness"; SpringPreload "Spring preload"; DamperCoef "Damping Coefficient"; DamperMaxExt "Maximum extension"; DampLimit_MaxK "Max_Stiffness"; DampLimit_MaxC "Max_Damping"; DampLimit_MaxTrans "Max_Transition"; DamperMinExt "Maximum compression"; DampLimit_MinK "Min_Stiffness"; DampLimit_MinC "Min_Damping"; DampLimit_MinTrans "Min_Transition"; PushSetupLength "Push"; ToeSetupLength "Toe"; UnsprungMass "Mass"; UnsprungMassCOG "COG"; RodThick "Rod Thickness"; RodDiam "Rod Diameter"
      mask help: Define a Simscape Multibody model of a double control arm independent suspension
      mask init:
      | dblAarmSuspOptim_init(gcb)
      mask Help:
      | -Geometry:
      |     +Suspension Coordinates: coordinates of the points that define a double wishbone suspension system. The coordinates must always correspond to the LEFT side. The variable (e.g. 'X') containing the data must be a row vector with the following values, in order:
      |       1-A-Arm Upper Front hardpoint. X([1 2 3])
      |       2-A-Arm Upper Rear. X([4 5 6])
      |       3-A-Arm Lower Front. X([7 8 9])
      |       4-A-Arm Lower Rear. X([10 11 12])
      |       5-A-Arm Upper Upright. X([13 14 15])
      |       6-A-Arm Lower Upright. X([16 17 18])
      |       7-Steering Rack. X([19 20 21])
      |       8-Steering Upright. X([22 23 24])
      |       9-Wheel Center. X([25 26 27])
      |       10-Wheel Plane normal vector. X([28 29 30])
      |       11-A-Arm Push Bracket. X([31 32 33])
      |       12-Bellcrank Push Bracket. X([34 35 36])
      |       13-Bellcrank Chassis Bracket. X([37 38 39])
      |       14-Bellcrank Damper Bracket. X([40 41 42])
      |       15-Damper Bracket. X([43 44 45])
      |       16-Bellcrank ARB Bracket. X(46:48)
      |       17-ARB end. X(49:51)
      |       18-ARB center. X(52:54)
      |     +It must be noted that in the model the bellcranks have the same dimensions as defined in the geometry, but the position and orientation is diferent. It is positioned in a vertical plane that contains the a-arm push bracket. The reason is to let the anti-roll bar be symmetric.
      | 
      | -Graphic: a CAD file can be specified to graphically represent some parts of the suspension. In order for the part to appear in its proper position, its reference axis and origin in the CAD file must be the same as the reference for the suspension points coordinates.
      mask TypeOptions side: Left | Right
      mask Callback side:
      | switch get_param(gcb,'side')
      |     case 'Left'
      |         set_param(gcb,'sideSign','1');
      |     case 'Right'
      |         set_param(gcb,'sideSign','-1');
      | end
      mask DialogControl DescGroupVar Prompt: Double Wishbone Suspension
      mask DialogControl SuspGeomTab Prompt: Geometry
      mask DialogControl X_original Tooltip: Variable containting suspension points coordinates
      mask DialogControl Container25 Prompt: A-arms geometry
      mask DialogControl enOptim Tooltip: Below parameters are used to modify the provided geometry. In this enable button is off no changes will be made
      mask DialogControl Container26 Prompt: Upper arm
      mask DialogControl Container27 Prompt: Lower arm
      mask DialogControl Container23 Prompt: Anti-roll bar
      mask DialogControl ARB_bcrnk Tooltip: Coordinates of the attachment point of the anti-roll bar to the bellcrank (mm)
      mask DialogControl Container8 Prompt: Wheel angles
      mask DialogControl Camber Tooltip: Camber angle in deg at reference ride height
      mask DialogControl Toe Tooltip: Toe angle in deg at reference ride height
      mask DialogControl Container22 Prompt: Assembly
      mask DialogControl BcrnkPos Tooltip: Degrees of rotation of follower frame respect to base frame of the bellcrank bracket joint for the inital position
      mask DialogControl DamperPos Tooltip: Degrees of rotation of follower frame respect to base frame of the bellcrank bracket joint for the inital position, specified as a rotation sequence X-Y-Z
      mask DialogControl ARB_On Tooltip: Select wheter the block displays connection for ARB or not
      mask DialogControl St_On Tooltip: Select wether the block displays steering input or not
      mask DialogControl SpringDampTab Prompt: Spring and Damper
      mask DialogControl SpringStiffness Tooltip: Spring stiffness in lb/inch
      mask DialogControl SpringPreload Tooltip: Spring preload in mm
      mask DialogControl DamperCoef Tooltip: Damping Coefficient in N/(mm/s)
      mask DialogControl Container9 Prompt: Limits
      mask DialogControl DamperMaxExt Tooltip: Maximum allowable extension of the damper, meassured between ball-joints (mm)
      mask DialogControl DampLimit_MaxK Tooltip: Stiffness when upper limit is reached in N/mm
      mask DialogControl DampLimit_MaxC Tooltip: Damping coefficient when upper limit is reached in N/(mm/s)
      mask DialogControl DampLimit_MaxTrans Tooltip: Transition region width between normal behavior and limit
      mask DialogControl DamperMinExt Tooltip: Minimum allowable extension (max compression) of the damper, meassured between ball-joints (mm)
      mask DialogControl DampLimit_MinK Tooltip: Stiffness when lower limit is reached in N/(mm/s)
      mask DialogControl DampLimit_MinC Tooltip: Damping coefficient when lower limit is reached in N/(mm/s)
      mask DialogControl DampLimit_MinTrans Tooltip: Transition region width between normal behavior and limit
      mask DialogControl Container13 Prompt: Setup
      mask DialogControl PushSetupLength Tooltip: Length adjustment of the rod ends in the push rod
      mask DialogControl ToeSetupLength Tooltip: Length adjustment of the rod ends in the toe/steering rod
      mask DialogControl Container19 Prompt: Mass
      mask DialogControl Container20 Prompt: Non-rotating unsprung mass
      mask DialogControl UnsprungMass Tooltip: Non rotating unsprung mass (kg)
      mask DialogControl UnsprungMassCOG Tooltip: Non-rotating unsprung mass center of gravity coordinates (mm)
      mask DialogControl Container17 Prompt: Graphic
      mask DialogControl internalParamContainer Prompt: Internal params
      mask Display: image('$imagefile'); % Use "Edit Image" on toolstrip to change.
  Selector1 : Selector  NumberOfDimensions=2  InputPortWidth=4  IndexOptions=Index vector (dialog),Select all  Indices=3,3  OutputSizes=1,1
  Selector2 : Selector  NumberOfDimensions=2  InputPortWidth=4  IndexOptions=Index vector (dialog),Select all  Indices=1,1  OutputSizes=1,1
  Selector3 : Selector  NumberOfDimensions=2  InputPortWidth=4  IndexOptions=Index vector (dialog),Select all  Indices=3,3  OutputSizes=1,1
  Selector4 : Selector  NumberOfDimensions=2  InputPortWidth=4  IndexOptions=Index vector (dialog),Select all  Indices=4,4  OutputSizes=1,1
  Selector5 : Selector  NumberOfDimensions=2  InputPortWidth=4  IndexOptions=Index vector (dialog),Select all  Indices=2,2  OutputSizes=1,1
  Selector6 : Selector  NumberOfDimensions=2  InputPortWidth=4  IndexOptions=Index vector (dialog),Select all  Indices=1,1  OutputSizes=1,1
  Selector7 : Selector  NumberOfDimensions=2  InputPortWidth=4  IndexOptions=Index vector (dialog),Select all  Indices=4,4  OutputSizes=1,1
  Selector8 : Selector  NumberOfDimensions=2  InputPortWidth=4  IndexOptions=Index vector (dialog),Select all  Indices=2,2  OutputSizes=1,1
  Sensors : Subsystem
  Steering rack : Subsystem
      settings: PortSchema="{\"entries\":[{\"content\":{\"sides\":[{\"content\":{\"connectorIds\":[\"LConn1\"]},\"type\":\"ConnectorPlacement.EquallySpacedRectSide\",\"uuid\":\"6dc6ed4f-bc40-436b-b956-95802cd9ab0b\"},{\"content\":{\"connectorIds\":[\"RConn1\"],\"side\":\"RIGHT\"},\"type\":\"ConnectorPlacement.EquallySpacedRectSide\",\"uuid\":\"bd6c321d-8aef-4dea-b6e1-aa7a472e67f8\"},{\"content\":{\"connectorIds\":[],\"side\":\"TOP\"},\"type\":\"ConnectorPlacement.EquallySpacedRectSide\",\"uuid\":\"c6af7ff4-db9d-47e0-b1fc-962e1cf0b8c4\"},{\"content\":{\"connectorIds\":[\"RConn2\",\"In1\"],\"side\":\"BOTTOM\"},\"type\":\"ConnectorPlacement.EquallySpacedRectSide\",\"uuid\":\"93e0e125-4db7-45ac-b4d6-39baf46eca45\"}]},\"type\":\"ConnectorPlacement.EquallySpacedConnector\",\"uuid\":\"ca57ac6f-7feb-47cf-9a03-b9bce30e5561\"}],\"packageUris\":[\"http://schema.mathworks.com/mf0/Connector/19700101\"],\"version\":\"1.0\"}"
  GroundRef : PMIOPort  Side=Right
  Info : Outport
  tag scope: FL_TireInfo=global, FR_TireInfo=global, RL_TireInfo=global, RR_TireInfo=global
  wires:
  Rack ratio/1 -> Steering rack/1(Steering input)
  StrngAng/1 -> Rack ratio/1
  Sensors/1(Info) -> Info/1
  F/1 -> Selector2/1
  BrkPrs1/1 -> Selector1/1
  AxlTrq1/1 -> Selector3/1
  M/1 -> Selector6/1
  Selector6/1 -> FL Wheel/2(M)
  Selector2/1 -> FL Wheel/1(F)
  Selector1/1 -> RL Wheel/1(F)
  Selector3/1 -> RL Wheel/2(M)
  Selector5/1 -> FR Wheel/1(F)
  BrkPrs2/1 -> Selector5/1
  Selector8/1 -> FR Wheel/2(M)
  AxlTrq2/1 -> Selector8/1
  Selector7/1 -> RR Wheel/2(M)
  Selector4/1 -> RR Wheel/1(F)
  BrkPrs3/1 -> Selector4/1
  AxlTrq3/1 -> Selector7/1
  FL Wheel/1(Info) -> [FL_TireInfo]
  FR Wheel/1(Info) -> [FR_TireInfo]
  RR Wheel/1(Info) -> [RR_TireInfo]
  RL Wheel/1(Info) -> [RL_TireInfo]
  connections:
  Rear Right Suspension/LConn1 -- ARB Rear/RConn2 -- Rear Left Suspension/LConn1 -- Chassis & Aero/LConn1 -- Connection Label6/LConn1 -- Cameras/RConn1 -- Front Right Suspension/LConn3 -- ARB Front/RConn2 -- Front Left Suspension/LConn3 -- Steering rack/RConn2
  ARB Rear/LConn1 -- Rear Left Suspension/LConn2
  Steering rack/LConn1 -- Front Left Suspension/LConn1
  RL Wheel/RConn1 -- Rear Left Suspension/RConn1
  Front Left Suspension/LConn2 -- ARB Front/LConn1
  Rear Right Suspension/LConn2 -- ARB Rear/RConn1
  Front Right Suspension/LConn2 -- ARB Front/RConn1
  FR Wheel/RConn1 -- Front Right Suspension/RConn1
  Front Right Suspension/LConn1 -- Steering rack/RConn1
  RR Wheel/RConn1 -- Rear Right Suspension/RConn1
  FL Wheel/RConn1 -- Front Left Suspension/RConn1
  Connection Label/LConn1 -- GroundRef/RConn1
